simulink model "IEEE_34_node_2024a"  (R2024a) (continued)
== system IEEE_34_node_2024a == (continued)
  888-890 : spsDistributedParametersLineLib/Distributed Parameters Line  Phases=3  Length=10560*ft2km  Frequency=Frequency  Resistance=R_300  Inductance=L_300  Capacitance=C_300  x1=[-1.418e-05 141.544961554255 0 0;-1.417e-05 141.544521033999 0 0;-1.416e-05 141.544080511733 0 0;-1.415e-05 141.543639987454 0 0;-1.414e-05 141.543199461164 0 0;-1.413e-05 141.542758932862 0 0;-1.412e-05 141.542318402549 0 0;-1.411e-05 141.541877870224 0 0;-1.41e-05 141.541437335887 0 0;-1.409e-05 141.540996799539 0 0;-1.408e-05 141.540556261179 0 0;-1.407e-05 141.540115720808 0 0;-1.406e-05 141.539675178425 0 0;-1.405e-05 141.53923463403 0 0;-1.404e-05 141.538794087624 0 0;-1.403e-05 141.538353539206 0 0;-1.402e-05 141.537912988777 0 0;-1.401e-05 141.537472436336 0 0;-1.4e-05 141.537031881883 0 0;-1.399e-05 141.536591325419 0 0;-1.398e-05 141.536150766943 0 0;-1.397e-05 141.535710206456 0 0;-1.396e-05 141.535269643957 0 0;-1.395e-05 141.534829079447 0 0;-1.394e-05 141.534388512925 0 0;-1.393e-05 141.533947944392 0 0;-1.392e-05 141.533507373847 0 0;-1.391e-05 141.533066801291 0 0;-1.39e-05 141.532626226723 0 0;-1.389e-05 141.532185650144 0 0;-1.388e-05 141.531745071553 0 0;-1.387e-05 141.531304490951 0 0;-1.386e-05 141.530863908337 0 0;-1.385e-05 141.530423323712 0 0;-1.384e-05 141.529982737075 0 0;-1.383e-05 141.529542148427 0 0;-1.382e-05 141.529101557767 0 0;-1.381e-05 141.528660965096 0 0;-1.38e-05 141.528220370414 0 0;-1.379e-05 141.52777977372 0 0;-1.378e-05 141.527339175014 0 0;-1.377e-05 141.526898574298 0 0;-1.376e-05 141.52645797157 0 0;-1.375e-05 141.52601736683 0 0;-1.374e-05 141.525576760079 0 0;-1.373e-05 141.525136151317 0 0;-1.372e-05 141.524695540543 0 0;-1.371e-05 141.524254927758 0 0;-1.37e-05 141.523814312961 0 0;-1.369e-05 141.523373696153 0 0;-1.368e-05 141.522933077334 0 0;-1.367e-05 141.522492456503 0 0;-1.366e-05 141.522051833661 0 0;-1.365e-05 141.521611208808 0 0;-1.364e-05 141.521170581943 0 0;-1.363e-05 141.520729953068 0 0;-1.362e-05 141.52028932218 0 0;-1.361e-05 141.519848689282 0 0;-1.36e-05 141.519408054372 0 0;-1.359e-05 141.51896741745 0 0;-1.358e-05 141.518526778518 0 0;-1.357e-05 141.518086137574 0 0;-1.356e-05 141.517645494619 0 0;-1.355e-05 141.517204849653 0 0;-1.354e-05 141.516764202675 0 0;-1.353e-05 141.516323553686 0 0;-1.352e-05 141.515882902686 0 0;-1.351e-05 141.515442249674 0 0;-1.35e-05 141.515001594652 0 0;-1.349e-05 141.514560937618 0 0;-1.348e-05 141.514120278572 0 0;-1.347e-05 141.513679617516 0 0;-1.346e-05 141.513238954448 0 0;-1.345e-05 141.512798289369 0 0;-1.344e-05 141.512357622279 0 0;-1.343e-05 141.511916953178 0 0;-1.342e-05 141.511476282066 0 0;-1.341e-05 141.511035608942 0 0;-1.34e-05 141.510594933807 0 0;-1.339e-05 141.510154256661 0 0;-1.338e-05 141.509713577504 0 0;-1.337e-05 141.509272896335 0 0;-1.336e-05 141.508832213156 0 0;-1.335e-05 141.508391527965 0 0;-1.334e-05 141.507950840763 0 0;-1.333e-05 141.50751015155 0 0;-1.332e-05 141.507069460326 0 0;-1.331e-05 141.506628767091 0 0;-1.33e-05 141.506188071844 0 0;-1.329e-05 141.505747374587 0 0;-1.328e-05 141.505306675318 0 0;-1.327e-05 141.504865974039 0 0;-1.326e-05 141.504425270748 0 0;-1.325e-05 141.503984565446 0 0;-1.324e-05 141.503543858133 0 0;-1.323e-05 141.503103148809 0 0;-1.322e-05 141.502662437474 0 0;-1.321e-05 141.502221724127 0 0;-1.32e-05 141.50178100877 0 0;-1.319e-05 141.501340291402 0 0;-1.318e-05 141.500899572022 0 0;-1.317e-05 141.500458850632 0 0;-1.316e-05 141.50001812723 0 0;-1.315e-05 141.499577401818 0 0;-1.314e-05 141.499136674394 0 0;-1.313e-05 141.49869594496 0 0;-1.312e-05 141.498255213514 0 0;-1.311e-05 141.497814480058 0 0;-1.31e-05 141.49737374459 0 0;-1.309e-05 141.496933007111 0 0;-1.308e-05 141.496492267622 0 0;-1.307e-05 141.496051526121 0 0;-1.306e-05 141.49561078261 0 0;-1.305e-05 141.495170037087 0 0;-1.304e-05 141.494729289554 0 0;-1.303e-05 141.494288540009 0 0;-1.302e-05 141.493847788454 0 0;-1.301e-05 141.493407034888 0 0;-1.3e-05 141.492966279311 0 0;-1.299e-05 141.492525521722 0 0;-1.298e-05 141.492084762123 0 0;-1.297e-05 141.491644000513 0 0;-1.296e-05 141.491203236892 0 0;-1.295e-05 141.490762471261 0 0;-1.294e-05 141.490321703618 0 0;-1.293e-05 ...(+75585 chars)]  x2=[-1.418e-05 140.065873089947 0 0;-1.417e-05 140.065351905099 0 0;-1.416e-05 140.06483071826 0 0;-1.415e-05 140.06430952943 0 0;-1.414e-05 140.06378833861 0 0;-1.413e-05 140.063267145799 0 0;-1.412e-05 140.062745950998 0 0;-1.411e-05 140.062224754206 0 0;-1.41e-05 140.061703555424 0 0;-1.409e-05 140.061182354651 0 0;-1.408e-05 140.060661151887 0 0;-1.407e-05 140.060139947133 0 0;-1.406e-05 140.059618740388 0 0;-1.405e-05 140.059097531653 0 0;-1.404e-05 140.058576320927 0 0;-1.403e-05 140.05805510821 0 0;-1.402e-05 140.057533893503 0 0;-1.401e-05 140.057012676806 0 0;-1.4e-05 140.056491458118 0 0;-1.399e-05 140.055970237439 0 0;-1.398e-05 140.05544901477 0 0;-1.397e-05 140.05492779011 0 0;-1.396e-05 140.05440656346 0 0;-1.395e-05 140.05388533482 0 0;-1.394e-05 140.053364104189 0 0;-1.393e-05 140.052842871567 0 0;-1.392e-05 140.052321636955 0 0;-1.391e-05 140.051800400353 0 0;-1.39e-05 140.05127916176 0 0;-1.389e-05 140.050757921176 0 0;-1.388e-05 140.050236678603 0 0;-1.387e-05 140.049715434039 0 0;-1.386e-05 140.049194187484 0 0;-1.385e-05 140.048672938939 0 0;-1.384e-05 140.048151688404 0 0;-1.383e-05 140.047630435878 0 0;-1.382e-05 140.047109181361 0 0;-1.381e-05 140.046587924855 0 0;-1.38e-05 140.046066666358 0 0;-1.379e-05 140.045545405871 0 0;-1.378e-05 140.045024143393 0 0;-1.377e-05 140.044502878925 0 0;-1.376e-05 140.043981612467 0 0;-1.375e-05 140.043460344018 0 0;-1.374e-05 140.042939073579 0 0;-1.373e-05 140.042417801149 0 0;-1.372e-05 140.04189652673 0 0;-1.371e-05 140.04137525032 0 0;-1.37e-05 140.040853971919 0 0;-1.369e-05 140.040332691529 0 0;-1.368e-05 140.039811409148 0 0;-1.367e-05 140.039290124777 0 0;-1.366e-05 140.038768838415 0 0;-1.365e-05 140.038247550064 0 0;-1.364e-05 140.037726259722 0 0;-1.363e-05 140.03720496739 0 0;-1.362e-05 140.036683673067 0 0;-1.361e-05 140.036162376755 0 0;-1.36e-05 140.035641078452 0 0;-1.359e-05 140.035119778159 0 0;-1.358e-05 140.034598475875 0 0;-1.357e-05 140.034077171602 0 0;-1.356e-05 140.033555865338 0 0;-1.355e-05 140.033034557084 0 0;-1.354e-05 140.03251324684 0 0;-1.353e-05 140.031991934606 0 0;-1.352e-05 140.031470620381 0 0;-1.351e-05 140.030949304167 0 0;-1.35e-05 140.030427985962 0 0;-1.349e-05 140.029906665767 0 0;-1.348e-05 140.029385343582 0 0;-1.347e-05 140.028864019407 0 0;-1.346e-05 140.028342693242 0 0;-1.345e-05 140.027821365086 0 0;-1.344e-05 140.027300034941 0 0;-1.343e-05 140.026778702805 0 0;-1.342e-05 140.026257368679 0 0;-1.341e-05 140.025736032564 0 0;-1.34e-05 140.025214694458 0 0;-1.339e-05 140.024693354362 0 0;-1.338e-05 140.024172012276 0 0;-1.337e-05 140.0236506682 0 0;-1.336e-05 140.023129322134 0 0;-1.335e-05 140.022607974078 0 0;-1.334e-05 140.022086624031 0 0;-1.333e-05 140.021565271995 0 0;-1.332e-05 140.021043917969 0 0;-1.331e-05 140.020522561953 0 0;-1.33e-05 140.020001203946 0 0;-1.329e-05 140.01947984395 0 0;-1.328e-05 140.018958481964 0 0;-1.327e-05 140.018437117988 0 0;-1.326e-05 140.017915752022 0 0;-1.325e-05 140.017394384066 0 0;-1.324e-05 140.016873014119 0 0;-1.323e-05 140.016351642183 0 0;-1.322e-05 140.015830268257 0 0;-1.321e-05 140.015308892341 0 0;-1.32e-05 140.014787514436 0 0;-1.319e-05 140.01426613454 0 0;-1.318e-05 140.013744752654 0 0;-1.317e-05 140.013223368779 0 0;-1.316e-05 140.012701982913 0 0;-1.315e-05 140.012180595058 0 0;-1.314e-05 140.011659205212 0 0;-1.313e-05 140.011137813377 0 0;-1.312e-05 140.010616419552 0 0;-1.311e-05 140.010095023737 0 0;-1.31e-05 140.009573625933 0 0;-1.309e-05 140.009052226138 0 0;-1.308e-05 140.008530824353 0 0;-1.307e-05 140.008009420579 0 0;-1.306e-05 140.007488014815 0 0;-1.305e-05 140.006966607061 0 0;-1.304e-05 140.006445197317 0 0;-1.303e-05 140.005923785584 0 0;-1.302e-05 140.005402371861 0 0;-1.301e-05 140.004880956147 0 0;-1.3e-05 140.004359538445 0 0;-1.299e-05 140.003838118752 0 0;-1.298e-05 140.003316697069 0 0;-1.297e-05 140.002795273397 0 0;-1.296e-05 140.002273847735 0 0;-1.295e-05 140.001752420084 0 0;-1.294e-05 140.001230990442 0 0;-1.293e-05 140.000709558811 0 ...(+75549 chars)]  x3=[-1.418e-05 3.13922854896028 0 0;-1.417e-05 3.13921848252525 0 0;-1.416e-05 3.13920841604562 0 0;-1.415e-05 3.13919834952136 0 0;-1.414e-05 3.1391882829525 0 0;-1.413e-05 3.13917821633901 0 0;-1.412e-05 3.13916814968092 0 0;-1.411e-05 3.13915808297821 0 0;-1.41e-05 3.13914801623088 0 0;-1.409e-05 3.13913794943894 0 0;-1.408e-05 3.13912788260239 0 0;-1.407e-05 3.13911781572122 0 0;-1.406e-05 3.13910774879544 0 0;-1.405e-05 3.13909768182504 0 0;-1.404e-05 3.13908761481003 0 0;-1.403e-05 3.13907754775041 0 0;-1.402e-05 3.13906748064617 0 0;-1.401e-05 3.13905741349732 0 0;-1.4e-05 3.13904734630386 0 0;-1.399e-05 3.13903727906579 0 0;-1.398e-05 3.1390272117831 0 0;-1.397e-05 3.1390171444558 0 0;-1.396e-05 3.13900707708388 0 0;-1.395e-05 3.13899700966736 0 0;-1.394e-05 3.13898694220622 0 0;-1.393e-05 3.13897687470047 0 0;-1.392e-05 3.13896680715011 0 0;-1.391e-05 3.13895673955514 0 0;-1.39e-05 3.13894667191555 0 0;-1.389e-05 3.13893660423136 0 0;-1.388e-05 3.13892653650255 0 0;-1.387e-05 3.13891646872913 0 0;-1.386e-05 3.1389064009111 0 0;-1.385e-05 3.13889633304846 0 0;-1.384e-05 3.13888626514121 0 0;-1.383e-05 3.13887619718935 0 0;-1.382e-05 3.13886612919288 0 0;-1.381e-05 3.1388560611518 0 0;-1.38e-05 3.1388459930661 0 0;-1.379e-05 3.1388359249358 0 0;-1.378e-05 3.13882585676089 0 0;-1.377e-05 3.13881578854137 0 0;-1.376e-05 3.13880572027724 0 0;-1.375e-05 3.1387956519685 0 0;-1.374e-05 3.13878558361514 0 0;-1.373e-05 3.13877551521719 0 0;-1.372e-05 3.13876544677462 0 0;-1.371e-05 3.13875537828744 0 0;-1.37e-05 3.13874530975565 0 0;-1.369e-05 3.13873524117926 0 0;-1.368e-05 3.13872517255826 0 0;-1.367e-05 3.13871510389265 0 0;-1.366e-05 3.13870503518243 0 0;-1.365e-05 3.1386949664276 0 0;-1.364e-05 3.13868489762816 0 0;-1.363e-05 3.13867482878412 0 0;-1.362e-05 3.13866475989547 0 0;-1.361e-05 3.13865469096221 0 0;-1.36e-05 3.13864462198435 0 0;-1.359e-05 3.13863455296187 0 0;-1.358e-05 3.13862448389479 0 0;-1.357e-05 3.13861441478311 0 0;-1.356e-05 3.13860434562682 0 0;-1.355e-05 3.13859427642592 0 0;-1.354e-05 3.13858420718041 0 0;-1.353e-05 3.1385741378903 0 0;-1.352e-05 3.13856406855558 0 0;-1.351e-05 3.13855399917626 0 0;-1.35e-05 3.13854392975233 0 0;-1.349e-05 3.13853386028379 0 0;-1.348e-05 3.13852379077065 0 0;-1.347e-05 3.1385137212129 0 0;-1.346e-05 3.13850365161055 0 0;-1.345e-05 3.13849358196359 0 0;-1.344e-05 3.13848351227203 0 0;-1.343e-05 3.13847344253586 0 0;-1.342e-05 3.13846337275509 0 0;-1.341e-05 3.13845330292971 0 0;-1.34e-05 3.13844323305973 0 0;-1.339e-05 3.13843316314515 0 0;-1.338e-05 3.13842309318596 0 0;-1.337e-05 3.13841302318216 0 0;-1.336e-05 3.13840295313377 0 0;-1.335e-05 3.13839288304077 0 0;-1.334e-05 3.13838281290316 0 0;-1.333e-05 3.13837274272096 0 0;-1.332e-05 3.13836267249414 0 0;-1.331e-05 3.13835260222273 0 0;-1.33e-05 3.13834253190671 0 0;-1.329e-05 3.13833246154609 0 0;-1.328e-05 3.13832239114087 0 0;-1.327e-05 3.13831232069104 0 0;-1.326e-05 3.13830225019662 0 0;-1.325e-05 3.13829217965759 0 0;-1.324e-05 3.13828210907395 0 0;-1.323e-05 3.13827203844572 0 0;-1.322e-05 3.13826196777288 0 0;-1.321e-05 3.13825189705545 0 0;-1.32e-05 3.13824182629341 0 0;-1.319e-05 3.13823175548677 0 0;-1.318e-05 3.13822168463553 0 0;-1.317e-05 3.13821161373968 0 0;-1.316e-05 3.13820154279924 0 0;-1.315e-05 3.13819147181419 0 0;-1.314e-05 3.13818140078455 0 0;-1.313e-05 3.1381713297103 0 0;-1.312e-05 3.13816125859146 0 0;-1.311e-05 3.13815118742801 0 0;-1.31e-05 3.13814111621996 0 0;-1.309e-05 3.13813104496731 0 0;-1.308e-05 3.13812097367007 0 0;-1.307e-05 3.13811090232822 0 0;-1.306e-05 3.13810083094178 0 0;-1.305e-05 3.13809075951073 0 0;-1.304e-05 3.13808068803508 0 0;-1.303e-05 3.13807061651484 0 0;-1.302e-05 3.13806054495 0 0;-1.301e-05 3.13805047334056 0 0;-1.3e-05 3.13804040168652 0 0;-1.299e-05 3.13803032998788 0 0;-1.298e-05 3.13802025824464 0 0;-1.297e-05 3.1380101864568 0 0;-1.296e-05 3.13800011462437 0 0;-1.295e-05 3.13799004274734 0 0;-1.294e-05 3.13797997082571 0 0;-1.293e-05 3.13796989885948 ...(+77940 chars)]  x4=[-1.418e-05 -2.79850851872357 0 0;-1.417e-05 -2.79849960961478 0 0;-1.416e-05 -2.79849070046621 0 0;-1.415e-05 -2.79848179127788 0 0;-1.414e-05 -2.79847288204977 0 0;-1.413e-05 -2.79846397278189 0 0;-1.412e-05 -2.79845506347424 0 0;-1.411e-05 -2.79844615412681 0 0;-1.41e-05 -2.79843724473961 0 0;-1.409e-05 -2.79842833531264 0 0;-1.408e-05 -2.7984194258459 0 0;-1.407e-05 -2.79841051633939 0 0;-1.406e-05 -2.7984016067931 0 0;-1.405e-05 -2.79839269720705 0 0;-1.404e-05 -2.79838378758122 0 0;-1.403e-05 -2.79837487791562 0 0;-1.402e-05 -2.79836596821025 0 0;-1.401e-05 -2.79835705846511 0 0;-1.4e-05 -2.79834814868019 0 0;-1.399e-05 -2.79833923885551 0 0;-1.398e-05 -2.79833032899106 0 0;-1.397e-05 -2.79832141908683 0 0;-1.396e-05 -2.79831250914284 0 0;-1.395e-05 -2.79830359915907 0 0;-1.394e-05 -2.79829468913554 0 0;-1.393e-05 -2.79828577907223 0 0;-1.392e-05 -2.79827686896916 0 0;-1.391e-05 -2.79826795882631 0 0;-1.39e-05 -2.7982590486437 0 0;-1.389e-05 -2.79825013842131 0 0;-1.388e-05 -2.79824122815916 0 0;-1.387e-05 -2.79823231785724 0 0;-1.386e-05 -2.79822340751555 0 0;-1.385e-05 -2.79821449713408 0 0;-1.384e-05 -2.79820558671286 0 0;-1.383e-05 -2.79819667625186 0 0;-1.382e-05 -2.79818776575109 0 0;-1.381e-05 -2.79817885521055 0 0;-1.38e-05 -2.79816994463025 0 0;-1.379e-05 -2.79816103401018 0 0;-1.378e-05 -2.79815212335034 0 0;-1.377e-05 -2.79814321265073 0 0;-1.376e-05 -2.79813430191135 0 0;-1.375e-05 -2.79812539113221 0 0;-1.374e-05 -2.7981164803133 0 0;-1.373e-05 -2.79810756945462 0 0;-1.372e-05 -2.79809865855617 0 0;-1.371e-05 -2.79808974761796 0 0;-1.37e-05 -2.79808083663998 0 0;-1.369e-05 -2.79807192562223 0 0;-1.368e-05 -2.79806301456472 0 0;-1.367e-05 -2.79805410346744 0 0;-1.366e-05 -2.79804519233039 0 0;-1.365e-05 -2.79803628115358 0 0;-1.364e-05 -2.798027369937 0 0;-1.363e-05 -2.79801845868065 0 0;-1.362e-05 -2.79800954738454 0 0;-1.361e-05 -2.79800063604866 0 0;-1.36e-05 -2.79799172467301 0 0;-1.359e-05 -2.7979828132576 0 0;-1.358e-05 -2.79797390180243 0 0;-1.357e-05 -2.79796499030749 0 0;-1.356e-05 -2.79795607877278 0 0;-1.355e-05 -2.79794716719831 0 0;-1.354e-05 -2.79793825558407 0 0;-1.353e-05 -2.79792934393007 0 0;-1.352e-05 -2.7979204322363 0 0;-1.351e-05 -2.79791152050277 0 0;-1.35e-05 -2.79790260872948 0 0;-1.349e-05 -2.79789369691642 0 0;-1.348e-05 -2.79788478506359 0 0;-1.347e-05 -2.797875873171 0 0;-1.346e-05 -2.79786696123865 0 0;-1.345e-05 -2.79785804926653 0 0;-1.344e-05 -2.79784913725465 0 0;-1.343e-05 -2.79784022520301 0 0;-1.342e-05 -2.7978313131116 0 0;-1.341e-05 -2.79782240098043 0 0;-1.34e-05 -2.79781348880949 0 0;-1.339e-05 -2.7978045765988 0 0;-1.338e-05 -2.79779566434834 0 0;-1.337e-05 -2.79778675205811 0 0;-1.336e-05 -2.79777783972813 0 0;-1.335e-05 -2.79776892735838 0 0;-1.334e-05 -2.79776001494887 0 0;-1.333e-05 -2.79775110249959 0 0;-1.332e-05 -2.79774219001056 0 0;-1.331e-05 -2.79773327748176 0 0;-1.33e-05 -2.7977243649132 0 0;-1.329e-05 -2.79771545230487 0 0;-1.328e-05 -2.79770653965679 0 0;-1.327e-05 -2.79769762696894 0 0;-1.326e-05 -2.79768871424134 0 0;-1.325e-05 -2.79767980147397 0 0;-1.324e-05 -2.79767088866684 0 0;-1.323e-05 -2.79766197581995 0 0;-1.322e-05 -2.79765306293329 0 0;-1.321e-05 -2.79764415000688 0 0;-1.32e-05 -2.79763523704071 0 0;-1.319e-05 -2.79762632403477 0 0;-1.318e-05 -2.79761741098908 0 0;-1.317e-05 -2.79760849790362 0 0;-1.316e-05 -2.79759958477841 0 0;-1.315e-05 -2.79759067161343 0 0;-1.314e-05 -2.7975817584087 0 0;-1.313e-05 -2.7975728451642 0 0;-1.312e-05 -2.79756393187995 0 0;-1.311e-05 -2.79755501855593 0 0;-1.31e-05 -2.79754610519216 0 0;-1.309e-05 -2.79753719178862 0 0;-1.308e-05 -2.79752827834533 0 0;-1.307e-05 -2.79751936486228 0 0;-1.306e-05 -2.79751045133947 0 0;-1.305e-05 -2.7975015377769 0 0;-1.304e-05 -2.79749262417457 0 0;-1.303e-05 -2.79748371053248 0 0;-1.302e-05 -2.79747479685063 0 0;-1.301e-05 -2.79746588312903 0 0;-1.3e-05 -2.79745696936767 0 0;-1.299e-05 -2.79744805556655 0 0;-1.298e-05 -2.79743914172567 0 0;-1.297e-05 -2.79743022784503 0 0 ...(+77016 chars)]  x5=1.41710191462344e-05  V1=ones(3,3)  V2=ones(3,3)  I1=ones(3,3)  I2=ones(3,3)  nHarmo=1  Measurements=None  Decoupling=off  VsMag0=0  VrMag0=0  VsAngle0=0  VrAngle0=0  IsMag0=0  IrMag0=0  IsAngle0=0  IrAngle0=0
      settings: LibrarySourceBlock=sps_lib/Power Grid Elements/Distributed Parameters Line
  890 : spsThreePhaseVIMeasurementLib/Three-Phase V-I Measurement  VoltageMeasurement=phase-to-ground  SetLabelV=on  LabelV=Vabc_890  Vpu=on  VpuLL=off  CurrentMeasurement=yes  SetLabelI=on  LabelI=Iabc_890  Ipu=on  Pbase=100e6  Vbase=Vnom1_ph_ph  OutputType=Magnitude-Angle  PhasorSimulation=off  PSBequivalent=0
  890 D-I : spsThreePhaseSeriesRLCLoadLib/Three-Phase Series RLC Load  LConnTagsString=A|B|C  Configuration=Delta  NominalVoltage=1000  Vabc=[1e3 1e3 1e3]  Vabcp=V_890*Vnom1  NominalFrequency=Frequency  UnbalancedPower=on  ActivePower=10e3  InductivePower=100  CapacitivePower=100  Pabc=[10e3 9e3 11e3]  QLabc=[100 90 110]  QCabc=[100 90 110]  Pabcp=Pa_890  QLabcp=Pp_890  QCabcp=Pn_890  Measurements=None  LoadType=constant I
  Ground{,1..5,8..15} (14 alike) : spsGroundLib/Ground  LConnTagsString=a
  Load Flow Bus : spsLoadFlowBusLib/Load Flow Bus  LConnTagsString=a|b|c  RConnTagsString=a|b|c  Phases=ABC  Connectors=on both sides  ID=800  Vbase=Vnom  Vref="[1.05  1.05  1.05]"  Vangle="[0  -120  120]"  VLF=1  VLFb=1  VLFc=1  angleLF=0  angleLFb=0  angleLFc=0
      settings: AttributesFormatString=%<ID>
  Load Flow Bus1 : spsLoadFlowBusLib/Load Flow Bus  LConnTagsString=a|b|c  RConnTagsString=a|b|c  Phases=ABC  Connectors=on both sides  ID=802  Vbase=Vnom  Vref=[1.0475 1.0484 1.0484]  Vangle=[-0.05 -120.07 119.95]  VLF=1  VLFb=1  VLFc=1  angleLF=0  angleLFb=0  angleLFc=0
      settings: AttributesFormatString=%<ID>
  Load Flow Bus10 : spsLoadFlowBusLib/Load Flow Bus  LConnTagsString=a  Phases=B  Connectors=on one side  ID=826  Vbase=Vnom  Vref=1.0156  Vangle=-122.94  VLF=1  VLFb=1  VLFc=1  angleLF=0  angleLFb=0  angleLFc=0
      settings: AttributesFormatString=%<ID>
  Load Flow Bus11 : spsLoadFlowBusLib/Load Flow Bus  LConnTagsString=a  RConnTagsString=a  Phases=A  Connectors=on both sides  ID=818  Vbase=Vnom  Vref=1.0163  Vangle=-2.27  VLF=1  VLFb=1  VLFc=1  angleLF=0  angleLFb=0  angleLFc=0
      settings: AttributesFormatString=%<ID>
  Load Flow Bus12 : spsLoadFlowBusLib/Load Flow Bus  LConnTagsString=a  RConnTagsString=a  Phases=A  Connectors=on both sides  ID=820  Vbase=Vnom  Vref=0.9926  Vangle=-2.32  VLF=1  VLFb=1  VLFc=1  angleLF=0  angleLFb=0  angleLFc=0
      settings: AttributesFormatString=%<ID>
  Load Flow Bus13 : spsLoadFlowBusLib/Load Flow Bus  LConnTagsString=a  Phases=A  Connectors=on one side  ID=822  Vbase=Vnom  Vref=0.9895  Vangle=-2.33  VLF=1  VLFb=1  VLFc=1  angleLF=0  angleLFb=0  angleLFc=0
      settings: AttributesFormatString=%<ID>
  Load Flow Bus14 : spsLoadFlowBusLib/Load Flow Bus  LConnTagsString=a|b|c  RConnTagsString=a|b|c  Phases=ABC  Connectors=on both sides  ID=828  Vbase=Vnom  Vref=[1.0074 1.0151 1.0109]  Vangle=[-2.38 -122.95 117.75]  VLF=1  VLFb=1  VLFc=1  angleLF=0  angleLFb=0  angleLFc=0
      settings: AttributesFormatString=%<ID>
  Load Flow Bus15 : spsLoadFlowBusLib/Load Flow Bus  LConnTagsString=a|b|c  RConnTagsString=a|b|c  Phases=ABC  Connectors=on both sides  ID=830  Vbase=Vnom  Vref=[0.9894 0.9982 0.9938]  Vangle=[-2.63 -123.39 117.25]  VLF=1  VLFb=1  VLFc=1  angleLF=0  angleLFb=0  angleLFc=0
      settings: AttributesFormatString=%<ID>
  Load Flow Bus16 : spsLoadFlowBusLib/Load Flow Bus  LConnTagsString=a|b|c  RConnTagsString=a|b|c  Phases=ABC  Connectors=on both sides  ID=854  Vbase=Vnom  Vref=[0.989 0.9978 0.9934]  Vangle=[-2.64 -123.4 117.24]  VLF=1  VLFb=1  VLFc=1  angleLF=0  angleLFb=0  angleLFc=0
      settings: AttributesFormatString=%<ID>
  Load Flow Bus17 : spsLoadFlowBusLib/Load Flow Bus  LConnTagsString=a  Phases=B  Connectors=on one side  ID=856  Vbase=Vnom  Vref=0.9977  Vangle=-123.41  VLF=1  VLFb=1  VLFc=1  angleLF=0  angleLFb=0  angleLFc=0
      settings: AttributesFormatString=%<ID>
  Load Flow Bus18 : spsLoadFlowBusLib/Load Flow Bus  LConnTagsString=a|b|c  RConnTagsString=a|b|c  Phases=ABC  Connectors=on both sides  ID=852  Vbase=Vnom  Vref=[0.9581 0.968 0.9637]  Vangle=[-3.11 -124.18 116.33]  VLF=1  VLFb=1  VLFc=1  angleLF=0  angleLFb=0  angleLFc=0
      settings: AttributesFormatString=%<ID>
  Load Flow Bus19 : spsLoadFlowBusLib/Load Flow Bus  LConnTagsString=a|b|c  RConnTagsString=a|b|c  Phases=ABC  Connectors=on both sides  ID=832  Vbase=Vnom  Vref=[1.0359 1.0345 1.036]  Vangle=[-3.11 -124.18 116.33]  VLF=1  VLFb=1  VLFc=1  angleLF=0  angleLFb=0  angleLFc=0
      settings: AttributesFormatString=%<ID>
  Load Flow Bus2 : spsLoadFlowBusLib/Load Flow Bus  LConnTagsString=a|b|c  RConnTagsString=a|b|c  Phases=ABC  Connectors=on both sides  ID=806  Vbase=Vnom  Vref=[1.0457 1.0474 1.0474]  Vangle=[-0.08 -120.11 119.92]  VLF=1  VLFb=1  VLFc=1  angleLF=0  angleLFb=0  angleLFc=0
      settings: AttributesFormatString=%<ID>
  Load Flow Bus20 : spsLoadFlowBusLib/Load Flow Bus  LConnTagsString=a|b|c  RConnTagsString=a|b|c  Phases=ABC  Connectors=on both sides  ID=888  Vbase=Vnom1  Vref=[0.9996 0.9983 1]  Vangle=[-4.64 -125.73 114.82]  VLF=1  VLFb=1  VLFc=1  angleLF=0  angleLFb=0  angleLFc=0
      settings: AttributesFormatString=%<ID>
  Load Flow Bus21 : spsLoadFlowBusLib/Load Flow Bus  LConnTagsString=a|b|c  RConnTagsString=a|b|c  Phases=ABC  Connectors=on both sides  ID=890  Vbase=Vnom1  Vref=[0.9167 0.9235 0.9177]  Vangle=[-5.19 -126.78 113.98]  VLF=1  VLFb=1  VLFc=1  angleLF=0  angleLFb=0  angleLFc=0
      settings: AttributesFormatString=%<ID>
  Load Flow Bus22 : spsLoadFlowBusLib/Load Flow Bus  LConnTagsString=a|b|c  RConnTagsString=a|b|c  Phases=ABC  Connectors=on both sides  ID=858  Vbase=Vnom  Vref=[1.0336 1.0322 1.0338]  Vangle=[-3.17 -124.28 116.22]  VLF=1  VLFb=1  VLFc=1  angleLF=0  angleLFb=0  angleLFc=0
      settings: AttributesFormatString=%<ID>
  Load Flow Bus23 : spsLoadFlowBusLib/Load Flow Bus  LConnTagsString=a  Phases=A  Connectors=on one side  ID=864  Vbase=Vnom  Vref=1.0336  Vangle=-3.17  VLF=1  VLFb=1  VLFc=1  angleLF=0  angleLFb=0  angleLFc=0
      settings: AttributesFormatString=%<ID>
  Load Flow Bus24 : spsLoadFlowBusLib/Load Flow Bus  LConnTagsString=a|b|c  RConnTagsString=a|b|c  Phases=ABC  Connectors=on both sides  ID=834  Vbase=Vnom  Vref=[1.0309 1.0295 1.0313]  Vangle=[-3.24 -124.39 116.09]  VLF=1  VLFb=1  VLFc=1  angleLF=0  angleLFb=0  angleLFc=0
      settings: AttributesFormatString=%<ID>
  Load Flow Bus25 : spsLoadFlowBusLib/Load Flow Bus  LConnTagsString=a|b|c  RConnTagsString=a|b|c  Phases=ABC  Connectors=on both sides  ID=860  Vbase=Vnom  Vref=[1.0305 1.0291 1.031]  Vangle=[-3.24 -124.39 116.09]  VLF=1  VLFb=1  VLFc=1  angleLF=0  angleLFb=0  angleLFc=0
      settings: AttributesFormatString=%<ID>
  Load Flow Bus26 : spsLoadFlowBusLib/Load Flow Bus  LConnTagsString=a|b|c  RConnTagsString=a|b|c  Phases=ABC  Connectors=on both sides  ID=836  Vbase=Vnom  Vref=[1.0303 1.0287 1.0308]  Vangle=[-3.23 -124.39 116.09]  VLF=1  VLFb=1  VLFc=1  angleLF=0  angleLFb=0  angleLFc=0
      settings: AttributesFormatString=%<ID>
  Load Flow Bus27 : spsLoadFlowBusLib/Load Flow Bus  LConnTagsString=a|b|c  RConnTagsString=a|b|c  Phases=ABC  Connectors=on both sides  ID=840  Vbase=Vnom  Vref=[1.0303 1.0287 1.0308]  Vangle=[-3.23 -124.39 116.09]  VLF=1  VLFb=1  VLFc=1  angleLF=0  angleLFb=0  angleLFc=0
      settings: AttributesFormatString=%<ID>
  Load Flow Bus29 : spsLoadFlowBusLib/Load Flow Bus  LConnTagsString=a  Phases=B  Connectors=on one side  ID=838  Vbase=Vnom  Vref=1.0285  Vangle=-124.39  VLF=1  VLFb=1  VLFc=1  angleLF=0  angleLFb=0  angleLFc=0
      settings: AttributesFormatString=%<ID>
  Load Flow Bus3 : spsLoadFlowBusLib/Load Flow Bus  LConnTagsString=a|b|c  RConnTagsString=a|b|c  Phases=ABC  Connectors=on both sides  ID=808  Vbase=Vnom  Vref=[1.0136 1.0296 1.0289]  Vangle=[-0.75 -120.95 119.3]  VLF=1  VLFb=1  VLFc=1  angleLF=0  angleLFb=0  angleLFc=0
      settings: AttributesFormatString=%<ID>
  Load Flow Bus30 : spsLoadFlowBusLib/Load Flow Bus  LConnTagsString=a|b|c  RConnTagsString=a|b|c  Phases=ABC  Connectors=on both sides  ID=842  Vbase=Vnom  Vref=[1.0309 1.0294 1.0313]  Vangle=[-3.25 -124.39 116.09]  VLF=1  VLFb=1  VLFc=1  angleLF=0  angleLFb=0  angleLFc=0
      settings: AttributesFormatString=%<ID>
  Load Flow Bus31 : spsLoadFlowBusLib/Load Flow Bus  LConnTagsString=a|b|c  RConnTagsString=a|b|c  Phases=ABC  Connectors=on both sides  ID=844  Vbase=Vnom  Vref=[1.0307 1.0291 1.0311]  Vangle=[-3.27 -124.42 116.06]  VLF=1  VLFb=1  VLFc=1  angleLF=0  angleLFb=0  angleLFc=0
      settings: AttributesFormatString=%<ID>
  Load Flow Bus32 : spsLoadFlowBusLib/Load Flow Bus  LConnTagsString=a|b|c  RConnTagsString=a|b|c  Phases=ABC  Connectors=on both sides  ID=846  Vbase=Vnom  Vref=[1.0309 1.0291 1.0313]  Vangle=[-3.32 -124.46 116.01]  VLF=1  VLFb=1  VLFc=1  angleLF=0  angleLFb=0  angleLFc=0
      settings: AttributesFormatString=%<ID>
  Load Flow Bus33 : spsLoadFlowBusLib/Load Flow Bus  LConnTagsString=a|b|c  RConnTagsString=a|b|c  Phases=ABC  Connectors=on both sides  ID=848  Vbase=Vnom  Vref=[1.031 1.0291 1.0314]  Vangle=[-3.32 -124.47 116]  VLF=1  VLFb=1  VLFc=1  angleLF=0  angleLFb=0  angleLFc=0
      settings: AttributesFormatString=%<ID>
  Load Flow Bus34 : spsLoadFlowBusLib/Load Flow Bus  LConnTagsString=a  Phases=A  Connectors=on one side  ID=open862  Vbase=Vnom  Vref=1.0303  Vangle=-3.23  VLF=1  VLFb=1  VLFc=1  angleLF=0  angleLFb=0  angleLFc=0
      settings: AttributesFormatString=%<ID>
  Load Flow Bus35 : spsLoadFlowBusLib/Load Flow Bus  LConnTagsString=a  Phases=C  Connectors=on one side  ID=open862  Vbase=Vnom  Vref=1.0308  Vangle=116.09  VLF=1  VLFb=1  VLFc=1  angleLF=0  angleLFb=0  angleLFc=0
      settings: AttributesFormatString=%<ID>
  Load Flow Bus36 : spsLoadFlowBusLib/Load Flow Bus  LConnTagsString=a  RConnTagsString=a  Phases=B  Connectors=on both sides  ID=862  Vbase=Vnom  Vref=1.0287  Vangle=-124.39  VLF=1  VLFb=1  VLFc=1  angleLF=0  angleLFb=0  angleLFc=0
      settings: AttributesFormatString=%<ID>
  Load Flow Bus4 : spsLoadFlowBusLib/Load Flow Bus  LConnTagsString=a|b|c  RConnTagsString=a|b|c  Phases=ABC  Connectors=on both sides  ID=812  Vbase=Vnom  Vref=[0.9763 1.01 1.0069]  Vangle=[-1.57 -121.92 118.59]  VLF=1  VLFb=1  VLFc=1  angleLF=0  angleLFb=0  angleLFc=0
      settings: AttributesFormatString=%<ID>
  Load Flow Bus5 : spsLoadFlowBusLib/Load Flow Bus  LConnTagsString=a|b|c  RConnTagsString=a|b|c  Phases=ABC  Connectors=on both sides  ID=814  Vbase=Vnom  Vref=[0.9467 0.9945 0.9893]  Vangle=[-2.26 -122.7 118.01]  VLF=1  VLFb=1  VLFc=1  angleLF=0  angleLFb=0  angleLFc=0
      settings: AttributesFormatString=%<ID>
  Load Flow Bus6 : spsLoadFlowBusLib/Load Flow Bus  LConnTagsString=a  Phases=B  Connectors=on one side  ID=810  Vbase=Vnom  Vref=1.0294  Vangle=-120.95  VLF=1  VLFb=1  VLFc=1  angleLF=0  angleLFb=0  angleLFc=0
      settings: AttributesFormatString=%<ID>
  Load Flow Bus7 : spsLoadFlowBusLib/Load Flow Bus  LConnTagsString=a|b|c  RConnTagsString=a|b|c  Phases=ABC  Connectors=on both sides  ID=850  Vbase=Vnom  Vref=[1.0176 1.0255 1.0203]  Vangle=[-2.26 -122.7 118.01]  VLF=1  VLFb=1  VLFc=1  angleLF=0  angleLFb=0  angleLFc=0
      settings: AttributesFormatString=%<ID>
  Load Flow Bus8 : spsLoadFlowBusLib/Load Flow Bus  LConnTagsString=a|b|c  RConnTagsString=a|b|c  Phases=ABC  Connectors=on both sides  ID=816  Vbase=Vnom  Vref=[1.0172 1.0253 1.02]  Vangle=[-2.26 -122.71 1.02]  VLF=1  VLFb=1  VLFc=1  angleLF=0  angleLFb=0  angleLFc=0
      settings: AttributesFormatString=%<ID>
  Load Flow Bus9 : spsLoadFlowBusLib/Load Flow Bus  LConnTagsString=a|b|c  RConnTagsString=a|b|c  Phases=ABC  Connectors=on both sides  ID=824  Vbase=Vnom  Vref=[1.0082 1.0158 1.0116]  Vangle=[-2.37 -122.94 117.76]  VLF=1  VLFb=1  VLFc=1  angleLF=0  angleLFb=0  angleLFc=0
      settings: AttributesFormatString=%<ID>
  Power-Flow : Subsystem
      mask Display: disp('Power-Flow\nResults')
  XF-10 24.9kV / 4.16kV : spsThreePhaseTransformerTwoWindingsLib/Three-Phase Transformer (Two Windings)  Winding1Connection=Delta (D1)  Winding2Connection=Yg  CoreType=Three single-phase transformers  SetSaturation=off  Hysteresis=off  DataFile='hysteresis'  SetInitialFlux=off  Measurements=None  UNITS=pu  NominalPower=[ 500e3 , 60 ]  Winding1=[ Vnom_ph_ph , 1e-6 , 0.0408 ]  Winding2=[ Vnom1_ph_ph , 1e-6 , 0.0408 ]  Rm=500  Lm=500  L0=0.5  Saturation=[ 0,0 ; 0.0024,1.2 ; 1.0,1.52 ]  InitialFluxes=[ 0.8 , -0.8 , 0.7 ]  BreakLoop=off  DiscreteSolver=Trapezoidal iterative  DataType=off  TransfoNumber=1
  powergui : sps_lib/powergui  Priority=1  SimulationMode=Discrete  SampleTime=1e-8  frequency=60  Iterations=50  frequencyindice=60  Pbase=100e3  ErrMax=0.0001  UnitsV=kV  UnitsW=kW  buscounter=0  FunctionMessages=off  echomessages=off  EnableUseOfTLC=off  CurrentSourceSwitches=off  DisableSnubberDevices=off  DisableRonSwitches=off  DisableVfSwitches=off  DisplayEquations=off  AutomaticDiscreteSolvers=off  SolverType=Tustin/Backward Euler (TBE)  Interpol=off  ExternalGateDelay=off  methode=off  Ts=100  x0status=blocks  SPID=off  SwTol=0  HookPort=off  NonlinearTolerance=1e-4  nMaxIteration=100  ContinueOnMaxIteration=off  RestoreLinks=warning  ResistiveCurrentMeasurement=off  Frange=[0:2:500]  Ylog=off  Xlog=on  ShowGrid=off  save=off  variable=ZData  structure=0  ZoomFFT=on  StartTime=0.0  cycles=1  DisplayStyle=1  fundamental=60  FreqAxis=off  MaxFrequency=1000  frequencyindicesteady=1  RmsSteady=1  display=off
  connections:
  800 gen/RConn1 -- Load Flow Bus/LConn1
  800 gen/RConn2 -- Load Flow Bus/LConn2
  800 gen/RConn3 -- Load Flow Bus/LConn3
  800-802/RConn1 -- Load Flow Bus1/LConn1
  800-802/RConn2 -- Load Flow Bus1/LConn2
  800-802/RConn3 -- Load Flow Bus1/LConn3
  802/LConn1 -- Load Flow Bus1/RConn1
  802/LConn2 -- Load Flow Bus1/RConn2
  802/LConn3 -- Load Flow Bus1/RConn3
  802-806/LConn1 -- 802/RConn1 -- 802 Yg PQ/LConn1
  802-806/LConn2 -- 802/RConn2 -- 802 Yg PQ/LConn2
  802-806/LConn3 -- 802/RConn3 -- 802 Yg PQ/LConn3
  Load Flow Bus2/LConn1 -- 802-806/RConn1 -- 806 Yg PQ/LConn1
  Load Flow Bus2/LConn2 -- 802-806/RConn2 -- 806 Yg PQ/LConn2
  Load Flow Bus2/LConn3 -- 806 Yg PQ/LConn3 -- 802-806/RConn3
  808/LConn2 -- Load Flow Bus3/RConn2
  808/LConn3 -- Load Flow Bus3/RConn3
  Load Flow Bus3/LConn3 -- 806-808/RConn3
  Load Flow Bus3/LConn1 -- 806-808/RConn1
  Load Flow Bus3/LConn2 -- 806-808/RConn2
  808/LConn1 -- Load Flow Bus3/RConn1
  Load Flow Bus4/LConn3 -- 808-812/RConn3
  Load Flow Bus4/LConn1 -- 808-812/RConn1
  Load Flow Bus4/LConn2 -- 808-812/RConn2
  808-812/LConn1 -- 808/RConn1
  808-812/LConn2 -- 808 Y-I/LConn1 -- 808-810/LConn1 -- 808/RConn2
  808-812/LConn3 -- 808/RConn3
  Load Flow Bus5/LConn3 -- 812-814/RConn3
  Load Flow Bus5/LConn1 -- 812-814/RConn1
  Load Flow Bus5/LConn2 -- 812-814/RConn2
  Load Flow Bus7/LConn1 -- 814-850/RConn1
  Load Flow Bus7/LConn2 -- 814-850/RConn2
  Load Flow Bus7/LConn3 -- 814-850/RConn3
  816/LConn3 -- Load Flow Bus8/RConn3
  816/LConn1 -- Load Flow Bus8/RConn1
  816/LConn2 -- Load Flow Bus8/RConn2
  Load Flow Bus8/LConn2 -- 850-816/RConn2
  Load Flow Bus8/LConn3 -- 850-816/RConn3
  Load Flow Bus8/LConn1 -- 850-816/RConn1
  Load Flow Bus9/LConn2 -- 824 D-I/LConn2 -- 816-824/RConn2
  824/LConn1 -- Load Flow Bus9/RConn1
  Load Flow Bus9/LConn1 -- 824 D-I/LConn1 -- 816-824/RConn1
  824/LConn2 -- Load Flow Bus9/RConn2
  824/LConn3 -- Load Flow Bus9/RConn3
  Load Flow Bus9/LConn3 -- 824 D-I/LConn3 -- 816-824/RConn3
  816-824/LConn1 -- 816-818/LConn1 -- 816 D-I/LConn1 -- 816/RConn1
  816-824/LConn2 -- 816 D-I/LConn2 -- 816/RConn2
  816-824/LConn3 -- 816 D-I/LConn3 -- 816/RConn3
  824-828/LConn1 -- 824 Y-PQ/LConn1 -- 824/RConn1
  824-828/LConn2 -- 824 Y-PQ/LConn2 -- 824-826/LConn1 -- 824 Y-I/LConn1 -- 824/RConn2
  824-828/LConn3 -- 824 Y-PQ/LConn3 -- 824/RConn3
  818 Y-Z/LConn1 -- 818-820/LConn1 -- Load Flow Bus11/RConn1
  820 Y-PQ/LConn1 -- 820-822/LConn1 -- Load Flow Bus12/RConn1
  820 Y-Z/LConn1 -- 818-820/RConn1 -- Load Flow Bus12/LConn1
  822 Y-PQ/LConn1 -- Load Flow Bus13/LConn1 -- 820-822/RConn1
  828/LConn2 -- Load Flow Bus14/RConn2
  Load Flow Bus14/LConn3 -- 824-828/RConn3 -- 828 Y-PQ/LConn3
  828/LConn1 -- Load Flow Bus14/RConn1
  Load Flow Bus14/LConn1 -- 828 Y-PQ/LConn1 -- 824-828/RConn1
  828/LConn3 -- Load Flow Bus14/RConn3
  Load Flow Bus14/LConn2 -- 824-828/RConn2 -- 828 Y-PQ/LConn2
  830 Y-PQ/LConn1 -- Load Flow Bus15/LConn1 -- 828-830/RConn1
  830 Y-PQ/LConn2 -- Load Flow Bus15/LConn2 -- 828-830/RConn2
  830 Y-PQ/LConn3 -- Load Flow Bus15/LConn3 -- 828-830/RConn3
  828-830/LConn1 -- 828 Y-PQ1/LConn1 -- 828/RConn1
  828-830/LConn2 -- 828 Y-PQ1/LConn2 -- 828/RConn2
  828-830/LConn3 -- 828 Y-PQ1/LConn3 -- 828/RConn3
  Load Flow Bus16/LConn1 -- 830-854/RConn1
  Load Flow Bus16/LConn2 -- 830-854/RConn2
  Load Flow Bus16/LConn3 -- 830-854/RConn3
  Load Flow Bus18/LConn1 -- 854-852/RConn1
  Load Flow Bus18/LConn3 -- 854-852/RConn3
  Load Flow Bus18/LConn2 -- 854-852/RConn2
  Load Flow Bus19/LConn3 -- 852-832/RConn3
  Load Flow Bus19/LConn2 -- 852-832/RConn2
  832/LConn1 -- Load Flow Bus19/RConn1
  Load Flow Bus19/LConn1 -- 852-832/RConn1
  832/LConn2 -- Load Flow Bus19/RConn2
  832/LConn3 -- Load Flow Bus19/RConn3
  Load Flow Bus16/RConn2 -- 854-856/LConn1 -- 854 Y-PQ/LConn1 -- 854-852/LConn2
  Load Flow Bus16/RConn3 -- 854-852/LConn3
  Load Flow Bus16/RConn1 -- 854-852/LConn1
  890/LConn1 -- Load Flow Bus21/RConn1
  890/LConn2 -- Load Flow Bus21/RConn2
  Load Flow Bus21/LConn1 -- 888-890/RConn1
  Load Flow Bus21/LConn2 -- 888-890/RConn2
  890/LConn3 -- Load Flow Bus21/RConn3
  Load Flow Bus21/LConn3 -- 888-890/RConn3
  890 D-I/LConn1 -- 890/RConn1
  890 D-I/LConn2 -- 890/RConn2
  890 D-I/LConn3 -- 890/RConn3
  858-834/LConn2 -- 858 D-PQ/LConn2 -- 858/RConn2
  Load Flow Bus22/LConn3 -- 858 D-Z/LConn3 -- 832-858/RConn3
  Load Flow Bus22/LConn1 -- 858 D-Z/LConn1 -- 832-858/RConn1
  858/LConn3 -- Load Flow Bus22/RConn3
  Load Flow Bus22/LConn2 -- 858 D-Z/LConn2 -- 832-858/RConn2
  858/LConn1 -- Load Flow Bus22/RConn1
  858-834/LConn1 -- 858 D-PQ/LConn1 -- 858-864/LConn1 -- 858 Y-PQ/LConn1 -- 858/RConn1
  858/LConn2 -- Load Flow Bus22/RConn2
  858-834/LConn3 -- 858 D-PQ/LConn3 -- 858/RConn3
  Load Flow Bus25/LConn3 -- 860 D-Z/LConn3 -- 834-860/RConn3
  834-860/LConn1 -- 834 D-Z/LConn1 -- 834-842/LConn1 -- 834/RConn1
  834/LConn2 -- Load Flow Bus24/RConn2
  860/LConn3 -- Load Flow Bus25/RConn3
  834/LConn3 -- Load Flow Bus24/RConn3
  860/LConn1 -- Load Flow Bus25/RConn1
  Load Flow Bus24/LConn2 -- 834 D-PQ/LConn2 -- 858-834/RConn2
  Load Flow Bus24/LConn1 -- 834 D-PQ/LConn1 -- 858-834/RConn1
  Load Flow Bus24/LConn3 -- 834 D-PQ/LConn3 -- 858-834/RConn3
  834/LConn1 -- Load Flow Bus24/RConn1
  Load Flow Bus25/LConn2 -- 860 D-Z/LConn2 -- 834-860/RConn2
  860/LConn2 -- Load Flow Bus25/RConn2
  834-860/LConn3 -- 834 D-Z/LConn3 -- 834-842/LConn3 -- 834/RConn3
  834-860/LConn2 -- 834 D-Z/LConn2 -- 834-842/LConn2 -- 834/RConn2
  Load Flow Bus25/LConn1 -- 860 D-Z/LConn1 -- 834-860/RConn1
  832 D-Z/LConn1 -- 832/RConn1 -- XF-10 24.9kV / 4.16kV/LConn1 -- 832-858/LConn1
  832 D-Z/LConn2 -- 832/RConn2 -- XF-10 24.9kV / 4.16kV/LConn2 -- 832-858/LConn2
  Load Flow Bus26/LConn1 -- 836 D-PQ/LConn1 -- 860-836/RConn1
  Load Flow Bus26/LConn2 -- 836 D-PQ/LConn2 -- 860-836/RConn2
  836/LConn3 -- Load Flow Bus26/RConn3
  836/LConn1 -- Load Flow Bus26/RConn1
  Load Flow Bus26/LConn3 -- 836 D-PQ/LConn3 -- 860-836/RConn3
  836/LConn2 -- Load Flow Bus26/RConn2
  860-836/LConn1 -- 860 D-PQ/LConn1 -- 860 Y-PQ/LConn1 -- 860/RConn1
  860-836/LConn2 -- 860 D-PQ/LConn2 -- 860 Y-PQ/LConn2 -- 860/RConn2
  860-836/LConn3 -- 860 D-PQ/LConn3 -- 860 Y-PQ/LConn3 -- 860/RConn3
  Load Flow Bus27/LConn1 -- 836-840/RConn1 -- 840 D-I/LConn1
  Load Flow Bus27/LConn2 -- 836-840/RConn2 -- 840 D-I/LConn2
  840/LConn1 -- Load Flow Bus27/RConn1
  840/LConn2 -- Load Flow Bus27/RConn2
  Load Flow Bus27/LConn3 -- 836-840/RConn3 -- 840 D-I/LConn3
  840/LConn3 -- Load Flow Bus27/RConn3
  836-840/LConn1 -- 836/RConn1 -- 836-862/LConn1 -- 836 D-I/LConn1
  836-840/LConn2 -- 836-862/LConn2 -- 836/RConn2 -- 836 D-I/LConn2
  836-840/LConn3 -- 836-862/LConn3 -- 836/RConn3 -- 836 D-I/LConn3
  840 Y-I/LConn1 -- 840/RConn1
  840 Y-I/LConn2 -- 840/RConn2
  840 Y-I/LConn3 -- 840/RConn3
  Load Flow Bus30/LConn1 -- 834-842/RConn1
  842/LConn2 -- Load Flow Bus30/RConn2
  842/LConn3 -- Load Flow Bus30/RConn3
  Load Flow Bus30/LConn2 -- 834-842/RConn2
  Load Flow Bus30/LConn3 -- 834-842/RConn3
  842/LConn1 -- Load Flow Bus30/RConn1
  Load Flow Bus31/LConn2 -- 844 Y-PQ/LConn2 -- 842-844/RConn2
  844/LConn2 -- Load Flow Bus31/RConn2
  Load Flow Bus31/LConn1 -- 844 Y-PQ/LConn1 -- 842-844/RConn1
  844/LConn1 -- Load Flow Bus31/RConn1
  842-844/LConn1 -- 842 Y-PQ/LConn1 -- 842/RConn1
  842-844/LConn2 -- 842 Y-PQ/LConn2 -- 842/RConn2
  842-844/LConn3 -- 842 Y-PQ/LConn3 -- 842/RConn3
  Load Flow Bus32/LConn2 -- 844-846/RConn2 -- 846 Y-PQ/LConn2
  846/LConn2 -- Load Flow Bus32/RConn2
  Load Flow Bus32/LConn1 -- 844-846/RConn1 -- 846 Y-PQ/LConn1
  846/LConn1 -- Load Flow Bus32/RConn1
  844-846/LConn1 -- 844 Y-PQ1/LConn1 -- 844 Y-Z1/LConn1 -- 844 Y-Z/LConn1 -- 844/RConn1
  844-846/LConn2 -- 844 Y-PQ1/LConn2 -- 844 Y-Z1/LConn2 -- 844 Y-Z/LConn2 -- 844/RConn2
  844-846/LConn3 -- 844 Y-PQ1/LConn3 -- 844 Y-Z1/LConn3 -- 844 Y-Z/LConn3 -- 844/RConn3
  Load Flow Bus33/LConn2 -- 848 Y-PQ/LConn2 -- 846-848/RConn2
  848/LConn2 -- Load Flow Bus33/RConn2
  Load Flow Bus33/LConn1 -- 848 Y-PQ/LConn1 -- 846-848/RConn1
  848/LConn1 -- Load Flow Bus33/RConn1
  846-848/LConn1 -- 846 Y-PQ1/LConn1 -- 846/RConn1
  846-848/LConn2 -- 846 Y-PQ1/LConn2 -- 846/RConn2
  846-848/LConn3 -- 846 Y-PQ1/LConn3 -- 846/RConn3
  848 D-PQ/LConn1 -- 848 D-Z/LConn1 -- 848/RConn1
  848 D-PQ/LConn2 -- 848 D-Z/LConn2 -- 848/RConn2
  848 D-PQ/LConn3 -- 848 D-Z/LConn3 -- 848/RConn3
  Load Flow Bus20/LConn1 -- XF-10 24.9kV / 4.16kV/RConn1
  Load Flow Bus20/LConn2 -- XF-10 24.9kV / 4.16kV/RConn2
  Load Flow Bus20/LConn3 -- XF-10 24.9kV / 4.16kV/RConn3
  826 Y-I/LConn1 -- Load Flow Bus10/LConn1 -- 824-826/RConn1
  856 Y-PQ/LConn1 -- Load Flow Bus17/LConn1 -- 854-856/RConn1
  838 Y-PQ/LConn1 -- 862-838/RConn1 -- Load Flow Bus29/LConn1
  Ground1/LConn1 -- 810 Y-I/RConn1
  Ground2/LConn1 -- 818 Y-Z/RConn1
  Ground3/LConn1 -- 820 Y-Z/RConn1
  Ground4/LConn1 -- 820 Y-PQ/RConn1
  Ground5/LConn1 -- 822 Y-PQ/RConn1
  862-838/LConn1 -- 862 Y-PQ/LConn1 -- Load Flow Bus36/RConn1
  Ground8/LConn1 -- 824 Y-I/RConn1
  Ground9/LConn1 -- 826 Y-I/RConn1
  842-844/RConn3 -- 844 Y-PQ/LConn3 -- Load Flow Bus31/LConn3
  Load Flow Bus31/RConn3 -- 844/LConn3
  844-846/RConn3 -- 846 Y-PQ/LConn3 -- Load Flow Bus32/LConn3
  Load Flow Bus32/RConn3 -- 846/LConn3
  846-848/RConn3 -- 848 Y-PQ/LConn3 -- Load Flow Bus33/LConn3
  Load Flow Bus33/RConn3 -- 848/LConn3
  Ground10/LConn1 -- 854 Y-PQ/RConn1
  Ground11/LConn1 -- 856 Y-PQ/RConn1
  Ground12/LConn1 -- 858 Y-PQ/RConn1
  Ground13/LConn1 -- 864 Y-PQ/RConn1
  864 Y-PQ/LConn1 -- 858-864/RConn1 -- Load Flow Bus23/LConn1
  Ground14/LConn1 -- 862 Y-PQ/RConn1
  Ground15/LConn1 -- 838 Y-PQ/RConn1
  Load Flow Bus/RConn1 -- 800-802/LConn1
  Load Flow Bus/RConn2 -- 800-802/LConn2
  Load Flow Bus/RConn3 -- 800-802/LConn3
  Load Flow Bus2/RConn1 -- 806-808/LConn1
  Load Flow Bus2/RConn2 -- 806-808/LConn2
  Load Flow Bus2/RConn3 -- 806-808/LConn3
  812-814/LConn1 -- Load Flow Bus4/RConn1
  812-814/LConn2 -- Load Flow Bus4/RConn2
  812-814/LConn3 -- Load Flow Bus4/RConn3
  814-850/LConn1 -- Load Flow Bus5/RConn1
  814-850/LConn2 -- Load Flow Bus5/RConn2
  814-850/LConn3 -- Load Flow Bus5/RConn3
  850-816/LConn1 -- Load Flow Bus7/RConn1
  850-816/LConn2 -- Load Flow Bus7/RConn2
  850-816/LConn3 -- Load Flow Bus7/RConn3
  830/LConn1 -- Load Flow Bus15/RConn1
  830/LConn2 -- Load Flow Bus15/RConn2
  830/LConn3 -- Load Flow Bus15/RConn3
  830-854/LConn1 -- 830 D-Z/LConn1 -- 830/RConn1
  830-854/LConn2 -- 830 D-Z/LConn2 -- 830/RConn2
  830-854/LConn3 -- 830 D-Z/LConn3 -- 830/RConn3
  852-832/LConn1 -- Load Flow Bus18/RConn1
  852-832/LConn2 -- Load Flow Bus18/RConn2
  852-832/LConn3 -- Load Flow Bus18/RConn3
  888-890/LConn1 -- Load Flow Bus20/RConn1
  888-890/LConn2 -- Load Flow Bus20/RConn2
  888-890/LConn3 -- Load Flow Bus20/RConn3
  808-810/RConn1 -- 810 Y-I/LConn1 -- Load Flow Bus6/LConn1
  816-818/RConn1 -- Load Flow Bus11/LConn1
  832 D-Z/LConn3 -- 832/RConn3 -- XF-10 24.9kV / 4.16kV/LConn3 -- 832-858/LConn3
  Ground/LConn1 -- 808 Y-I/RConn1
  Load Flow Bus36/LConn1 -- 836-862/RConn2
  Load Flow Bus34/LConn1 -- 836-862/RConn1
  Load Flow Bus35/LConn1 -- 836-862/RConn3
